simulink model "code_replacement_example"  (R2024b)
settings: Solver=FixedStepAuto  StartTime=0.0  StopTime=10.0  RelTol=1e-3
settings Model: Creator=a0499511  LastModifiedBy=a0499511
settings OptimizationCC: DefaultParameterBehavior=Inlined  EnhancedBackFolding=on  PassReuseOutputArgsAs=Individual arguments  ZeroExternalMemoryAtStartup=off  ZeroInternalMemoryAtStartup=off  MaxStackSize=64  OptimizeBlockOrder=speed  DifferentSizesBufferReuse=on  OptimizationCustomize=off  EfficientTunableParamExpr=on
settings DebuggingCC: ParameterTunabilityLossMsg=error
settings HardwareCC: ProdBitPerPointer=32  ProdBitPerSizeT=32  ProdBitPerPtrDiffT=32  ProdLargestAtomicInteger=Long  ProdLargestAtomicFloat=Double  ProdWordSize=32  ProdHWDeviceType=ARM Compatible->ARM Cortex
settings SFSimCC: MATLABDynamicMemAlloc=off
settings RTWCC: Description=Embedded Coder  SystemTargetFile=ert.tlc  HardwareBoard=AM263PX LP  TemplateMakefile=ert_default_tmf  Toolchain=TI ARM Clang Compiler AM263Px | gmake
settings CodeAppCC: ForceParamTrailComments=on  IgnoreCustomStorageClasses=off  OperatorAnnotations=on  SimulinkDataObjDesc=on  SFDataObjDesc=on  InsertBlockDesc=on
settings ERTTargetCC: CodeReplacementLibrary=AM263P_TMU  SupportContinuousTime=on  ExtModeTransport=3  ExtModeStaticAlloc=on  ExtModeMexFile=ext_xcp  ExtModeIntrfLevel=Level2 - Open  ERTCustomFileTemplate=AM263x_codertarget_file_process.tlc

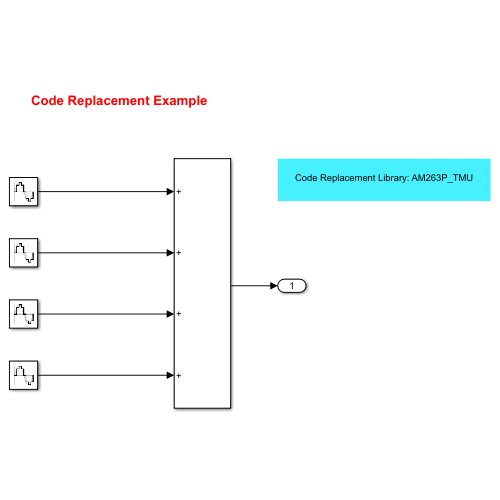
[thumbnail: Simulink's stored preview of the top-level diagram]
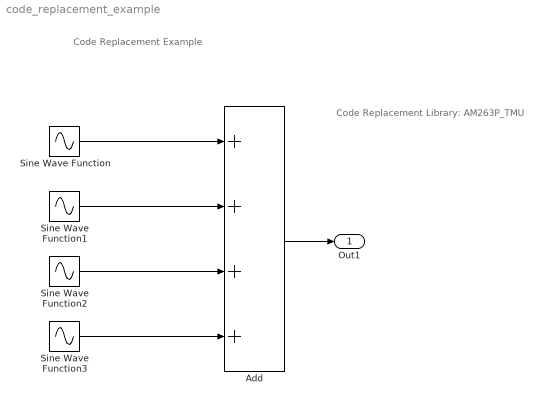
== system code_replacement_example ==
  note: Code Replacement Library: AM263P_TMU
  note: Code Replacement Example
  Add : Sum  Inputs=++++
  Sine Wave Function : Sin  Amplitude=46  Frequency=2*pi*50  SampleTime=7e-6
  Sine Wave Function1 : Sin  Amplitude=230*sqrt(2)  Frequency=2*pi*50  SampleTime=7e-6
  Sine Wave Function2 : Sin  Amplitude=30  Frequency=2*pi*50*3  SampleTime=7e-6
  Sine Wave Function3 : Sin  Amplitude=15  Frequency=2*pi*50*5  SampleTime=7e-6
  Out1 : Outport
  wires:
  Sine Wave Function/1 -> Add/1
  Sine Wave Function1/1 -> Add/2
  Sine Wave Function2/1 -> Add/3
  Sine Wave Function3/1 -> Add/4
  Add/1 -> Out1/1
